simulink model "ACC_scenario_007_cs"  (Simulink 7.6)
settings: Solver=ode5  SolverMode=SingleTasking  StartTime=0.0  StopTime=inf  FixedStep=1/100  RelTol=1e-3
PreLoadFcn:
  | check_NUTS_defined;
  | 
  | assignin('base',['model_closed_' bdroot],false);addpath('../includes_ACC');
InitFcn:
  | PreScanExperimentInitFcn(bdroot)
StopFcn:
  | PreScanExperimentStopFcn(bdroot)
undefined symbols (not defined in the file): DiscIntNeverNeededParam, x_ini_VRML, y_ini_VRML, z_ini_VRML
libraries: PreScanSimulinkLibrary x47, simulink x27, PreScanUsefulBlocks x15, simulink_extras x13, simulink_need_slupdate x1
(part 3 of 25)

== system ACC_scenario_007_cs/HighwayOverhang_11 ==
  note: Resources
      |
      | 1: InformationSign\MatrixSign_GreenArrow.png: MatrixSign GreenArrow
      | 2: InformationSign\TASS_logo.jpeg: TASS logo
      | 3: InformationSign\SEARCH_Logo.png: SEARCH Logo
      | 4: InformationSign\TheFuture.jpg: TheFuture
      | 5: InformationSign\NoParking.jpg: NoParking
      | 6: InformationSign\dont_even_think.jpg: dont even think
      | 7: InformationSign\sharp_edges.jpg: sharp edges
      | 8: InformationSign\tacos.jpg: tacos
      | 9: InformationSign\cthulhuwarn.jpg: cthulhuwarn
      | 10: InformationSign\road_surprises.jpg: road surprises
      | 11: InformationSign\sign1.jpg: sign1
      | 12: InformationSign\sign2.jpg: sign2
      | 13: InformationSign\sign3.jpg: sign3
      | 14: InformationSign\sign4.jpg: sign4
      | 15: InformationSign\sign5.JPG: sign5
      | 16: InformationSign\sign7.JPG: sign7
      | 17: InformationSign\sign10.png: sign10
      | 18: InformationSign\sign31.jpg: sign31
      | 19: InformationSign\sign32.jpg: sign32
  note: Resources
  Ground_1 : Ground
  Ground_2 : Ground
  VISUMatrixSign_Mux : Subsystem  Tag=MuxMatrixSign
      settings: DeleteFcn=PreScanBlockDeleteFcn;
      mask Type: PreScan Mux Block:
  VISUMatrixSign_HighwayOverhang_11 : Outport  Tag=VisuMatrixSignOutport  SampleTime=1/100
  wires:
  VISUMatrixSign_Mux/1(MatrixSigns) -> VISUMatrixSign_HighwayOverhang_11/1
  Ground_1/1 -> VISUMatrixSign_Mux/1(InformationPlate_13)
  Ground_2/1 -> VISUMatrixSign_Mux/2(InformationPlate_14)

== system ACC_scenario_007_cs/HighwayOverhang_12 ==
  note: Resources
      |
      | 1: InformationSign\MatrixSign_GreenArrow.png: MatrixSign GreenArrow
      | 2: InformationSign\TASS_logo.jpeg: TASS logo
      | 3: InformationSign\SEARCH_Logo.png: SEARCH Logo
      | 4: InformationSign\TheFuture.jpg: TheFuture
      | 5: InformationSign\NoParking.jpg: NoParking
      | 6: InformationSign\dont_even_think.jpg: dont even think
      | 7: InformationSign\sharp_edges.jpg: sharp edges
      | 8: InformationSign\tacos.jpg: tacos
      | 9: InformationSign\cthulhuwarn.jpg: cthulhuwarn
      | 10: InformationSign\road_surprises.jpg: road surprises
      | 11: InformationSign\sign1.jpg: sign1
      | 12: InformationSign\sign2.jpg: sign2
      | 13: InformationSign\sign3.jpg: sign3
      | 14: InformationSign\sign4.jpg: sign4
      | 15: InformationSign\sign5.JPG: sign5
      | 16: InformationSign\sign7.JPG: sign7
      | 17: InformationSign\sign10.png: sign10
      | 18: InformationSign\sign31.jpg: sign31
      | 19: InformationSign\sign32.jpg: sign32
  note: Resources
  Ground_1 : Ground
  Ground_2 : Ground
  VISUMatrixSign_Mux : Subsystem  Tag=MuxMatrixSign
      settings: DeleteFcn=PreScanBlockDeleteFcn;
      mask Type: PreScan Mux Block:
  VISUMatrixSign_HighwayOverhang_12 : Outport  Tag=VisuMatrixSignOutport  SampleTime=1/100
  wires:
  VISUMatrixSign_Mux/1(MatrixSigns) -> VISUMatrixSign_HighwayOverhang_12/1
  Ground_1/1 -> VISUMatrixSign_Mux/1(InformationPlate_15)
  Ground_2/1 -> VISUMatrixSign_Mux/2(InformationPlate_16)

== system ACC_scenario_007_cs/HighwayOverhang_2 ==
  note: Resources
      |
      | 1: InformationSign\MatrixSign_GreenArrow.png: MatrixSign GreenArrow
      | 2: InformationSign\TASS_logo.jpeg: TASS logo
      | 3: InformationSign\SEARCH_Logo.png: SEARCH Logo
      | 4: InformationSign\TheFuture.jpg: TheFuture
      | 5: InformationSign\NoParking.jpg: NoParking
      | 6: InformationSign\dont_even_think.jpg: dont even think
      | 7: InformationSign\sharp_edges.jpg: sharp edges
      | 8: InformationSign\tacos.jpg: tacos
      | 9: InformationSign\cthulhuwarn.jpg: cthulhuwarn
      | 10: InformationSign\road_surprises.jpg: road surprises
      | 11: InformationSign\sign1.jpg: sign1
      | 12: InformationSign\sign2.jpg: sign2
      | 13: InformationSign\sign3.jpg: sign3
      | 14: InformationSign\sign4.jpg: sign4
      | 15: InformationSign\sign5.JPG: sign5
      | 16: InformationSign\sign7.JPG: sign7
      | 17: InformationSign\sign10.png: sign10
      | 18: InformationSign\sign31.jpg: sign31
      | 19: InformationSign\sign32.jpg: sign32
  note: Resources
  Ground_{1..4} (4 alike) : Ground
  VISUMatrixSignLights_Mux : Subsystem  Tag=MuxMatrixSignLights
      settings: DeleteFcn=PreScanBlockDeleteFcn;
      mask Type: PreScan Mux Block:
  VISUMatrixSign_Mux : Subsystem  Tag=MuxMatrixSign
      settings: DeleteFcn=PreScanBlockDeleteFcn;
      mask Type: PreScan Mux Block:
  VISUMatrixSign_HighwayOverhang_2 : Outport  Tag=VisuMatrixSignOutport  SampleTime=1/100
  VISUMatrixSignLights_HighwayOverhang_2 : Outport  Tag=VisuMatrixSignLightsOutport  Port=2  SampleTime=1/100
  wires:
  VISUMatrixSign_Mux/1(MatrixSigns) -> VISUMatrixSign_HighwayOverhang_2/1
  VISUMatrixSignLights_Mux/1(MatrixSignLights) -> VISUMatrixSignLights_HighwayOverhang_2/1
  Ground_1/1 -> VISUMatrixSignLights_Mux/1(MatrixSign_4)
  Ground_2/1 -> VISUMatrixSignLights_Mux/2(MatrixSign_5)
  Ground_3/1 -> VISUMatrixSign_Mux/1(MatrixSign_4)
  Ground_4/1 -> VISUMatrixSign_Mux/2(MatrixSign_5)

== system ACC_scenario_007_cs/HighwayOverhang_3 ==
  note: Resources
      |
      | 1: InformationSign\MatrixSign_GreenArrow.png: MatrixSign GreenArrow
      | 2: InformationSign\TASS_logo.jpeg: TASS logo
      | 3: InformationSign\SEARCH_Logo.png: SEARCH Logo
      | 4: InformationSign\TheFuture.jpg: TheFuture
      | 5: InformationSign\NoParking.jpg: NoParking
      | 6: InformationSign\dont_even_think.jpg: dont even think
      | 7: InformationSign\sharp_edges.jpg: sharp edges
      | 8: InformationSign\tacos.jpg: tacos
      | 9: InformationSign\cthulhuwarn.jpg: cthulhuwarn
      | 10: InformationSign\road_surprises.jpg: road surprises
      | 11: InformationSign\sign1.jpg: sign1
      | 12: InformationSign\sign2.jpg: sign2
      | 13: InformationSign\sign3.jpg: sign3
      | 14: InformationSign\sign4.jpg: sign4
      | 15: InformationSign\sign5.JPG: sign5
      | 16: InformationSign\sign7.JPG: sign7
      | 17: InformationSign\sign10.png: sign10
      | 18: InformationSign\sign31.jpg: sign31
      | 19: InformationSign\sign32.jpg: sign32
  note: Resources
  Ground_{1..4} (4 alike) : Ground
  VISUMatrixSignLights_Mux : Subsystem  Tag=MuxMatrixSignLights
      settings: DeleteFcn=PreScanBlockDeleteFcn;
      mask Type: PreScan Mux Block:
  VISUMatrixSign_Mux : Subsystem  Tag=MuxMatrixSign
      settings: DeleteFcn=PreScanBlockDeleteFcn;
      mask Type: PreScan Mux Block:
  VISUMatrixSign_HighwayOverhang_3 : Outport  Tag=VisuMatrixSignOutport  SampleTime=1/100
  VISUMatrixSignLights_HighwayOverhang_3 : Outport  Tag=VisuMatrixSignLightsOutport  Port=2  SampleTime=1/100
  wires:
  VISUMatrixSign_Mux/1(MatrixSigns) -> VISUMatrixSign_HighwayOverhang_3/1
  VISUMatrixSignLights_Mux/1(MatrixSignLights) -> VISUMatrixSignLights_HighwayOverhang_3/1
  Ground_1/1 -> VISUMatrixSignLights_Mux/1(MatrixSign_7)
  Ground_2/1 -> VISUMatrixSignLights_Mux/2(MatrixSign_8)
  Ground_3/1 -> VISUMatrixSign_Mux/1(MatrixSign_7)
  Ground_4/1 -> VISUMatrixSign_Mux/2(MatrixSign_8)

== system ACC_scenario_007_cs/HighwayOverhang_4 ==
  note: Resources
      |
      | 1: InformationSign\MatrixSign_GreenArrow.png: MatrixSign GreenArrow
      | 2: InformationSign\TASS_logo.jpeg: TASS logo
      | 3: InformationSign\SEARCH_Logo.png: SEARCH Logo
      | 4: InformationSign\TheFuture.jpg: TheFuture
      | 5: InformationSign\NoParking.jpg: NoParking
      | 6: InformationSign\dont_even_think.jpg: dont even think
      | 7: InformationSign\sharp_edges.jpg: sharp edges
      | 8: InformationSign\tacos.jpg: tacos
      | 9: InformationSign\cthulhuwarn.jpg: cthulhuwarn
      | 10: InformationSign\road_surprises.jpg: road surprises
      | 11: InformationSign\sign1.jpg: sign1
      | 12: InformationSign\sign2.jpg: sign2
      | 13: InformationSign\sign3.jpg: sign3
      | 14: InformationSign\sign4.jpg: sign4
      | 15: InformationSign\sign5.JPG: sign5
      | 16: InformationSign\sign7.JPG: sign7
      | 17: InformationSign\sign10.png: sign10
      | 18: InformationSign\sign31.jpg: sign31
      | 19: InformationSign\sign32.jpg: sign32
  note: Resources
  Ground_{1..4} (4 alike) : Ground
  VISUMatrixSignLights_Mux : Subsystem  Tag=MuxMatrixSignLights
      settings: DeleteFcn=PreScanBlockDeleteFcn;
      mask Type: PreScan Mux Block:
  VISUMatrixSign_Mux : Subsystem  Tag=MuxMatrixSign
      settings: DeleteFcn=PreScanBlockDeleteFcn;
      mask Type: PreScan Mux Block:
  VISUMatrixSign_HighwayOverhang_4 : Outport  Tag=VisuMatrixSignOutport  SampleTime=1/100
  VISUMatrixSignLights_HighwayOverhang_4 : Outport  Tag=VisuMatrixSignLightsOutport  Port=2  SampleTime=1/100
  wires:
  VISUMatrixSign_Mux/1(MatrixSigns) -> VISUMatrixSign_HighwayOverhang_4/1
  VISUMatrixSignLights_Mux/1(MatrixSignLights) -> VISUMatrixSignLights_HighwayOverhang_4/1
  Ground_1/1 -> VISUMatrixSignLights_Mux/1(MatrixSign_10)
  Ground_2/1 -> VISUMatrixSignLights_Mux/2(MatrixSign_11)
  Ground_3/1 -> VISUMatrixSign_Mux/1(MatrixSign_10)
  Ground_4/1 -> VISUMatrixSign_Mux/2(MatrixSign_11)

== system ACC_scenario_007_cs/HighwayOverhang_5 ==
  note: Resources
      |
      | 1: InformationSign\MatrixSign_GreenArrow.png: MatrixSign GreenArrow
      | 2: InformationSign\TASS_logo.jpeg: TASS logo
      | 3: InformationSign\SEARCH_Logo.png: SEARCH Logo
      | 4: InformationSign\TheFuture.jpg: TheFuture
      | 5: InformationSign\NoParking.jpg: NoParking
      | 6: InformationSign\dont_even_think.jpg: dont even think
      | 7: InformationSign\sharp_edges.jpg: sharp edges
      | 8: InformationSign\tacos.jpg: tacos
      | 9: InformationSign\cthulhuwarn.jpg: cthulhuwarn
      | 10: InformationSign\road_surprises.jpg: road surprises
      | 11: InformationSign\sign1.jpg: sign1
      | 12: InformationSign\sign2.jpg: sign2
      | 13: InformationSign\sign3.jpg: sign3
      | 14: InformationSign\sign4.jpg: sign4
      | 15: InformationSign\sign5.JPG: sign5
      | 16: InformationSign\sign7.JPG: sign7
      | 17: InformationSign\sign10.png: sign10
      | 18: InformationSign\sign31.jpg: sign31
      | 19: InformationSign\sign32.jpg: sign32
  note: Resources
  Ground_1 : Ground
  Ground_2 : Ground
  VISUMatrixSign_Mux : Subsystem  Tag=MuxMatrixSign
      settings: DeleteFcn=PreScanBlockDeleteFcn;
      mask Type: PreScan Mux Block:
  VISUMatrixSign_HighwayOverhang_5 : Outport  Tag=VisuMatrixSignOutport  SampleTime=1/100
  wires:
  VISUMatrixSign_Mux/1(MatrixSigns) -> VISUMatrixSign_HighwayOverhang_5/1
  Ground_1/1 -> VISUMatrixSign_Mux/1(InformationPlate_1)
  Ground_2/1 -> VISUMatrixSign_Mux/2(InformationPlate_2)

== system ACC_scenario_007_cs/HighwayOverhang_6 ==
  note: Resources
      |
      | 1: InformationSign\MatrixSign_GreenArrow.png: MatrixSign GreenArrow
      | 2: InformationSign\TASS_logo.jpeg: TASS logo
      | 3: InformationSign\SEARCH_Logo.png: SEARCH Logo
      | 4: InformationSign\TheFuture.jpg: TheFuture
      | 5: InformationSign\NoParking.jpg: NoParking
      | 6: InformationSign\dont_even_think.jpg: dont even think
      | 7: InformationSign\sharp_edges.jpg: sharp edges
      | 8: InformationSign\tacos.jpg: tacos
      | 9: InformationSign\cthulhuwarn.jpg: cthulhuwarn
      | 10: InformationSign\road_surprises.jpg: road surprises
      | 11: InformationSign\sign1.jpg: sign1
      | 12: InformationSign\sign2.jpg: sign2
      | 13: InformationSign\sign3.jpg: sign3
      | 14: InformationSign\sign4.jpg: sign4
      | 15: InformationSign\sign5.JPG: sign5
      | 16: InformationSign\sign7.JPG: sign7
      | 17: InformationSign\sign10.png: sign10
      | 18: InformationSign\sign31.jpg: sign31
      | 19: InformationSign\sign32.jpg: sign32
  note: Resources
  Ground_1 : Ground
  Ground_2 : Ground
  VISUMatrixSign_Mux : Subsystem  Tag=MuxMatrixSign
      settings: DeleteFcn=PreScanBlockDeleteFcn;
      mask Type: PreScan Mux Block:
  VISUMatrixSign_HighwayOverhang_6 : Outport  Tag=VisuMatrixSignOutport  SampleTime=1/100
  wires:
  VISUMatrixSign_Mux/1(MatrixSigns) -> VISUMatrixSign_HighwayOverhang_6/1
  Ground_1/1 -> VISUMatrixSign_Mux/1(InformationPlate_3)
  Ground_2/1 -> VISUMatrixSign_Mux/2(InformationPlate_4)

== system ACC_scenario_007_cs/HighwayOverhang_7 ==
  note: Resources
      |
      | 1: InformationSign\MatrixSign_GreenArrow.png: MatrixSign GreenArrow
      | 2: InformationSign\TASS_logo.jpeg: TASS logo
      | 3: InformationSign\SEARCH_Logo.png: SEARCH Logo
      | 4: InformationSign\TheFuture.jpg: TheFuture
      | 5: InformationSign\NoParking.jpg: NoParking
      | 6: InformationSign\dont_even_think.jpg: dont even think
      | 7: InformationSign\sharp_edges.jpg: sharp edges
      | 8: InformationSign\tacos.jpg: tacos
      | 9: InformationSign\cthulhuwarn.jpg: cthulhuwarn
      | 10: InformationSign\road_surprises.jpg: road surprises
      | 11: InformationSign\sign1.jpg: sign1
      | 12: InformationSign\sign2.jpg: sign2
      | 13: InformationSign\sign3.jpg: sign3
      | 14: InformationSign\sign4.jpg: sign4
      | 15: InformationSign\sign5.JPG: sign5
      | 16: InformationSign\sign7.JPG: sign7
      | 17: InformationSign\sign10.png: sign10
      | 18: InformationSign\sign31.jpg: sign31
      | 19: InformationSign\sign32.jpg: sign32
  note: Resources
  Ground_1 : Ground
  Ground_2 : Ground
  VISUMatrixSign_Mux : Subsystem  Tag=MuxMatrixSign
      settings: DeleteFcn=PreScanBlockDeleteFcn;
      mask Type: PreScan Mux Block:
  VISUMatrixSign_HighwayOverhang_7 : Outport  Tag=VisuMatrixSignOutport  SampleTime=1/100
  wires:
  VISUMatrixSign_Mux/1(MatrixSigns) -> VISUMatrixSign_HighwayOverhang_7/1
  Ground_1/1 -> VISUMatrixSign_Mux/1(InformationPlate_9)
  Ground_2/1 -> VISUMatrixSign_Mux/2(InformationPlate_10)

== system ACC_scenario_007_cs/HighwayOverhang_8 ==
  note: Resources
      |
      | 1: InformationSign\MatrixSign_GreenArrow.png: MatrixSign GreenArrow
      | 2: InformationSign\TASS_logo.jpeg: TASS logo
      | 3: InformationSign\SEARCH_Logo.png: SEARCH Logo
      | 4: InformationSign\TheFuture.jpg: TheFuture
      | 5: InformationSign\NoParking.jpg: NoParking
      | 6: InformationSign\dont_even_think.jpg: dont even think
      | 7: InformationSign\sharp_edges.jpg: sharp edges
      | 8: InformationSign\tacos.jpg: tacos
      | 9: InformationSign\cthulhuwarn.jpg: cthulhuwarn
      | 10: InformationSign\road_surprises.jpg: road surprises
      | 11: InformationSign\sign1.jpg: sign1
      | 12: InformationSign\sign2.jpg: sign2
      | 13: InformationSign\sign3.jpg: sign3
      | 14: InformationSign\sign4.jpg: sign4
      | 15: InformationSign\sign5.JPG: sign5
      | 16: InformationSign\sign7.JPG: sign7
      | 17: InformationSign\sign10.png: sign10
      | 18: InformationSign\sign31.jpg: sign31
      | 19: InformationSign\sign32.jpg: sign32
  note: Resources
  Ground_1 : Ground
  Ground_2 : Ground
  VISUMatrixSign_Mux : Subsystem  Tag=MuxMatrixSign
      settings: DeleteFcn=PreScanBlockDeleteFcn;
      mask Type: PreScan Mux Block:
  VISUMatrixSign_HighwayOverhang_8 : Outport  Tag=VisuMatrixSignOutport  SampleTime=1/100
  wires:
  VISUMatrixSign_Mux/1(MatrixSigns) -> VISUMatrixSign_HighwayOverhang_8/1
  Ground_1/1 -> VISUMatrixSign_Mux/1(InformationPlate_11)
  Ground_2/1 -> VISUMatrixSign_Mux/2(InformationPlate_12)

== system ACC_scenario_007_cs/HighwayOverhang_9 ==
  note: Resources
      |
      | 1: InformationSign\MatrixSign_GreenArrow.png: MatrixSign GreenArrow
      | 2: InformationSign\TASS_logo.jpeg: TASS logo
      | 3: InformationSign\SEARCH_Logo.png: SEARCH Logo
      | 4: InformationSign\TheFuture.jpg: TheFuture
      | 5: InformationSign\NoParking.jpg: NoParking
      | 6: InformationSign\dont_even_think.jpg: dont even think
      | 7: InformationSign\sharp_edges.jpg: sharp edges
      | 8: InformationSign\tacos.jpg: tacos
      | 9: InformationSign\cthulhuwarn.jpg: cthulhuwarn
      | 10: InformationSign\road_surprises.jpg: road surprises
      | 11: InformationSign\sign1.jpg: sign1
      | 12: InformationSign\sign2.jpg: sign2
      | 13: InformationSign\sign3.jpg: sign3
      | 14: InformationSign\sign4.jpg: sign4
      | 15: InformationSign\sign5.JPG: sign5
      | 16: InformationSign\sign7.JPG: sign7
      | 17: InformationSign\sign10.png: sign10
      | 18: InformationSign\sign31.jpg: sign31
      | 19: InformationSign\sign32.jpg: sign32
  note: Resources
  Ground_1 : Ground
  Ground_2 : Ground
  VISUMatrixSign_Mux : Subsystem  Tag=MuxMatrixSign
      settings: DeleteFcn=PreScanBlockDeleteFcn;
      mask Type: PreScan Mux Block:
  VISUMatrixSign_HighwayOverhang_9 : Outport  Tag=VisuMatrixSignOutport  SampleTime=1/100
  wires:
  VISUMatrixSign_Mux/1(MatrixSigns) -> VISUMatrixSign_HighwayOverhang_9/1
  Ground_1/1 -> VISUMatrixSign_Mux/1(InformationPlate_5)
  Ground_2/1 -> VISUMatrixSign_Mux/2(InformationPlate_6)

== system ACC_scenario_007_cs/Lightpost_1 ==

== system ACC_scenario_007_cs/Lightpost_19 ==

== system ACC_scenario_007_cs/Lightpost_2 ==

== system ACC_scenario_007_cs/Lightpost_21 ==

== system ACC_scenario_007_cs/Lightpost_23 ==

== system ACC_scenario_007_cs/Lightpost_24 ==

== system ACC_scenario_007_cs/Lightpost_25 ==

== system ACC_scenario_007_cs/Lightpost_26 ==

== system ACC_scenario_007_cs/Lightpost_27 ==

== system ACC_scenario_007_cs/Lightpost_28 ==

== system ACC_scenario_007_cs/Lightpost_29 ==

== system ACC_scenario_007_cs/Lightpost_3 ==

== system ACC_scenario_007_cs/Lightpost_30 ==

== system ACC_scenario_007_cs/Lightpost_31 ==

== system ACC_scenario_007_cs/Lightpost_32 ==

== system ACC_scenario_007_cs/Lightpost_33 ==

== system ACC_scenario_007_cs/Lightpost_34 ==

== system ACC_scenario_007_cs/Lightpost_35 ==

== system ACC_scenario_007_cs/Lightpost_36 ==

== system ACC_scenario_007_cs/Lightpost_37 ==

== system ACC_scenario_007_cs/Lightpost_4 ==

== system ACC_scenario_007_cs/Lightpost_52 ==

== system ACC_scenario_007_cs/Lightpost_53 ==

== system ACC_scenario_007_cs/Lightpost_54 ==

== system ACC_scenario_007_cs/Lightpost_55 ==

== system ACC_scenario_007_cs/Lightpost_56 ==

== system ACC_scenario_007_cs/Lightpost_57 ==

== system ACC_scenario_007_cs/Lightpost_58 ==

== system ACC_scenario_007_cs/Lightpost_59 ==

== system ACC_scenario_007_cs/Lightpost_60 ==

== system ACC_scenario_007_cs/Lightpost_61 ==

== system ACC_scenario_007_cs/Lightpost_62 ==

== system ACC_scenario_007_cs/Lightpost_63 ==

== system ACC_scenario_007_cs/Lightpost_64 ==

== system ACC_scenario_007_cs/Lightpost_65 ==

== system ACC_scenario_007_cs/Lightpost_66 ==

== system ACC_scenario_007_cs/Lightpost_67 ==

== system ACC_scenario_007_cs/Lightpost_68 ==

== system ACC_scenario_007_cs/Nissan_Cabstar_1 ==
  SELF_Nissan_Cabstar_1 : Inport  Tag=SELFInport
  Ground_{1..20} (20 alike) : Ground
  SELF_Demux : Subsystem  Tag=DemuxSELF
      settings: DeleteFcn=PreScanBlockDeleteFcn;
      mask Type: PreScan Demux Block:
      mask Display:
      | port_label('output',1,'x [m]')
      | port_label('output',2,'y [m]')
      | port_label('output',3,'z [m]','texmode','on')
      | port_label('output',4,'Rotx [deg]','texmode','on')
      | port_label('output',5,'Roty [deg]','texmode','on')
      | port_label('output',6,'Rotz [deg]','texmode','on')
      | port_label('output',7,'GPS lat [deg]','texmode','on')
      | port_label('output',8,'GPS long [deg]','texmode','on')
      | port_label('output',9,'GPS alt [m]','texmode','on')
      | port_label('output',10,'Velocity [m/s]')
      | port_label('output',11,'Heading [deg]')
      | port_label('output',12,'Yaw rate [deg/s]')
  Terminator_{1..11} (11 alike) : Terminator
  Trajectories : Subsystem  Tag=Trajectories
      settings: DeleteFcn=PreScanBlockDeleteFcn;
      mask Type: PreScan Source Block: Trajectories
      mask Description:
      | The Trajectory block contains the trajectories defined in the PreScan Experiment Editor. The output of the trajectory block is the (vehicle) State.
      | 
      | For more information, please click Help.
      mask Help: eval('openHelp(312)')
      mask Display: image(imread('Trajectory.png'));
  VISULightSource_Mux : Subsystem  Tag=MuxLightSource
      settings: DeleteFcn=PreScanBlockDeleteFcn;
      mask Type: PreScan Mux Block:
  VISUSteer_Mux : Subsystem  Tag=MuxSteer
      settings: DeleteFcn=PreScanBlockDeleteFcn;
      mask Type: PreScan Mux Block:
  VISUWheels_Mux : Subsystem  Tag=MuxWheels  mask(PreScan Mux Block:){TimeStep=1/100}
      mask prompts: TimeStep "Auto Assigned: Time Step [s]"
      settings: DeleteFcn=PreScanBlockDeleteFcn;
  STATE_Nissan_Cabstar_1 : Outport  Tag=StateOutport
  VISULightSource_Nissan_Cabstar_1 : Outport  Tag=VisuLightsOutport  Port=2  SampleTime=1/100
  VISUSteer_Nissan_Cabstar_1 : Outport  Tag=VisuSteerOutport  Port=3  SampleTime=1/100
  VISUWheels_Nissan_Cabstar_1 : Outport  Tag=VisuWheelsOutport  Port=4  SampleTime=1/100
  wires:
  SELF_Nissan_Cabstar_1/1 -> SELF_Demux/1(SELF)
  VISULightSource_Mux/1(Lights) -> VISULightSource_Nissan_Cabstar_1/1
  VISUSteer_Mux/1(Steer) -> VISUSteer_Nissan_Cabstar_1/1
  VISUWheels_Mux/1(Wheels) -> VISUWheels_Nissan_Cabstar_1/1
  Trajectories/1(State) -> STATE_Nissan_Cabstar_1/1
  SELF_Demux/10(velocity [m/s]) -> VISUWheels_Mux/3(Velocity [m/s])
  SELF_Demux/1(x [m]) -> Terminator_1/1
  SELF_Demux/2(y [m]) -> Terminator_2/1
  SELF_Demux/3(z [m]) -> Terminator_3/1
  SELF_Demux/4(rotx [deg]) -> Terminator_4/1
  SELF_Demux/5(roty [deg]) -> Terminator_5/1
  SELF_Demux/6(rotz [deg]) -> Terminator_6/1
  SELF_Demux/7(GPS lat [deg]) -> Terminator_7/1
  SELF_Demux/8(GPS long [deg]) -> Terminator_8/1
  SELF_Demux/9(GPS alt [m]) -> Terminator_9/1
  SELF_Demux/11(heading [deg]) -> Terminator_10/1
  SELF_Demux/12(yaw rate [deg/s]) -> Terminator_11/1
  Ground_1/1 -> VISULightSource_Mux/1(IndicatorLightsLeft)
  Ground_2/1 -> VISULightSource_Mux/2(IndicatorLightsRight)
  Ground_3/1 -> VISULightSource_Mux/3(FogLightRearLeft)
  Ground_4/1 -> VISULightSource_Mux/4(FogLightRearRight)
  Ground_5/1 -> VISULightSource_Mux/5(FogLightFrontLeft)
  Ground_6/1 -> VISULightSource_Mux/6(FogLightFrontRight)
  Ground_7/1 -> VISULightSource_Mux/7(MainLightRearLeft)
  Ground_8/1 -> VISULightSource_Mux/8(MainLightRearRight)
  Ground_9/1 -> VISULightSource_Mux/9(MainLightFrontLeft)
  Ground_10/1 -> VISULightSource_Mux/10(MainLightFrontRight)
  Ground_11/1 -> VISULightSource_Mux/11(BrakeLightLeft)
  Ground_12/1 -> VISULightSource_Mux/12(BrakeLightRight)
  Ground_13/1 -> VISUSteer_Mux/1(Column [deg])
  Ground_14/1 -> VISUSteer_Mux/2(Steer [deg])
  Ground_15/1 -> VISUWheels_Mux/1(Steer Angle Front [deg])
  Ground_16/1 -> VISUWheels_Mux/2(Steer Angle Rear [deg])
  Ground_17/1 -> VISUWheels_Mux/4(zDisp FL [m])
  Ground_18/1 -> VISUWheels_Mux/5(zDisp FR [m])
  Ground_19/1 -> VISUWheels_Mux/6(zDisp RL [m])
  Ground_20/1 -> VISUWheels_Mux/7(zDisp RR [m])

== system ACC_scenario_007_cs/SELF_Actros_1860_1 ==
  Selector : Selector  IndexMode=Zero-based  IndexOptions=Starting index (dialog)  Indices=PortIndex  OutputSizes=PortWidth(1)
  U : Outport
  wires:
  Selector/1 -> U/1
  [SimCoreSensors] -> Selector/1

== system ACC_scenario_007_cs/SELF_Actros_2541_1 ==
  Selector : Selector  IndexMode=Zero-based  IndexOptions=Starting index (dialog)  Indices=PortIndex  OutputSizes=PortWidth(1)
  U : Outport
  wires:
  Selector/1 -> U/1
  [SimCoreSensors] -> Selector/1

== system ACC_scenario_007_cs/SELF_Actros_2541_2 ==
  Selector : Selector  IndexMode=Zero-based  IndexOptions=Starting index (dialog)  Indices=PortIndex  OutputSizes=PortWidth(1)
  U : Outport
  wires:
  Selector/1 -> U/1
  [SimCoreSensors] -> Selector/1

== system ACC_scenario_007_cs/SELF_Actros_2541_3 ==
  Selector : Selector  IndexMode=Zero-based  IndexOptions=Starting index (dialog)  Indices=PortIndex  OutputSizes=PortWidth(1)
  U : Outport
  wires:
  Selector/1 -> U/1
  [SimCoreSensors] -> Selector/1

== system ACC_scenario_007_cs/SELF_Audi_A8_1 ==
  Selector : Selector  IndexMode=Zero-based  IndexOptions=Starting index (dialog)  Indices=PortIndex  OutputSizes=PortWidth(1)
  U : Outport
  wires:
  Selector/1 -> U/1
  [SimCoreSensors] -> Selector/1

== system ACC_scenario_007_cs/SELF_BMW_X5_1 ==
  Selector : Selector  IndexMode=Zero-based  IndexOptions=Starting index (dialog)  Indices=PortIndex  OutputSizes=PortWidth(1)
  U : Outport
  wires:
  Selector/1 -> U/1
  [SimCoreSensors] -> Selector/1

== system ACC_scenario_007_cs/SELF_BMW_X5_2 ==
  Selector : Selector  IndexMode=Zero-based  IndexOptions=Starting index (dialog)  Indices=PortIndex  OutputSizes=PortWidth(1)
  U : Outport
  wires:
  Selector/1 -> U/1
  [SimCoreSensors] -> Selector/1

== system ACC_scenario_007_cs/SELF_Citroen_C3_1 ==
  Selector : Selector  IndexMode=Zero-based  IndexOptions=Starting index (dialog)  Indices=PortIndex  OutputSizes=PortWidth(1)
  U : Outport
  wires:
  Selector/1 -> U/1
  [SimCoreSensors] -> Selector/1

== system ACC_scenario_007_cs/SELF_ContainerTrailer_DoubleAxle_1 ==
  Selector : Selector  IndexMode=Zero-based  IndexOptions=Starting index (dialog)  Indices=PortIndex  OutputSizes=PortWidth(1)
  U : Outport
  wires:
  Selector/1 -> U/1
  [SimCoreSensors] -> Selector/1

== system ACC_scenario_007_cs/SELF_Fiat_Bravo_1 ==
  Selector : Selector  IndexMode=Zero-based  IndexOptions=Starting index (dialog)  Indices=PortIndex  OutputSizes=PortWidth(1)
  U : Outport
  wires:
  Selector/1 -> U/1
  [SimCoreSensors] -> Selector/1

== system ACC_scenario_007_cs/SELF_Nissan_Cabstar_1 ==
  Selector : Selector  IndexMode=Zero-based  IndexOptions=Starting index (dialog)  Indices=PortIndex  OutputSizes=PortWidth(1)
  U : Outport
  wires:
  Selector/1 -> U/1
  [SimCoreSensors] -> Selector/1

== system ACC_scenario_007_cs/SELF_Sphere_1 ==
  Selector : Selector  IndexMode=Zero-based  IndexOptions=Starting index (dialog)  Indices=PortIndex  OutputSizes=PortWidth(1)
  U : Outport
  wires:
  Selector/1 -> U/1
  [SimCoreSensors] -> Selector/1

== system ACC_scenario_007_cs/SELF_Toyota_Yaris_1 ==
  Selector : Selector  IndexMode=Zero-based  IndexOptions=Starting index (dialog)  Indices=PortIndex  OutputSizes=PortWidth(1)
  U : Outport
  wires:
  Selector/1 -> U/1
  [SimCoreSensors] -> Selector/1

== system ACC_scenario_007_cs/STATE_Actros_1860_1 ==
  State : Inport  SampleTime=TimeStep
  Acceleration : Subsystem  mask(ToSensorWorld){PortName=Acceleration_Actros_1860_1, PortIndex=1240, PortWidth=3, TimeStep=1/100}
      mask prompts: PortName "Port Name [-]"; PortIndex "Index [-]"; PortWidth "Port Dimensions [-]"; TimeStep "Upload Period [s]"
      mask help: Sends signal(s) from Simulink to the Sensor World
      mask Help:
      | Sends signal(s) from Simulink to the Sensor World.
      | 
      | - Port Name [-]: specifies the name of the output terminal of the Simulink block.
      | - Index [-]: specifies the start position in the output vector sent to the Sensor World
      | - Port dimensions [-]: specifies the width (number of scalars) of the output port
      | - Upload period [s]: specifies the upload period (in seconds) of the port.
      mask Display: port_label('input', 1, PortName)
  Demux : Demux  Outputs=5
  Position : Subsystem  mask(ToSensorWorld){PortName=Position_Actros_1860_1, PortIndex=1234, PortWidth=3, TimeStep=1/100}
      mask prompts: PortName "Port Name [-]"; PortIndex "Index [-]"; PortWidth "Port Dimensions [-]"; TimeStep "Upload Period [s]"
      mask help: Sends signal(s) from Simulink to the Sensor World
      mask Help:
      | Sends signal(s) from Simulink to the Sensor World.
      | 
      | - Port Name [-]: specifies the name of the output terminal of the Simulink block.
      | - Index [-]: specifies the start position in the output vector sent to the Sensor World
      | - Port dimensions [-]: specifies the width (number of scalars) of the output port
      | - Upload period [s]: specifies the upload period (in seconds) of the port.
      mask Display: port_label('input', 1, PortName)
  RPY : Subsystem  mask(ToSensorWorld){PortName=RPY_Actros_1860_1, PortIndex=1243, PortWidth=3, TimeStep=1/100}
      mask prompts: PortName "Port Name [-]"; PortIndex "Index [-]"; PortWidth "Port Dimensions [-]"; TimeStep "Upload Period [s]"
      mask help: Sends signal(s) from Simulink to the Sensor World
      mask Help:
      | Sends signal(s) from Simulink to the Sensor World.
      | 
      | - Port Name [-]: specifies the name of the output terminal of the Simulink block.
      | - Index [-]: specifies the start position in the output vector sent to the Sensor World
      | - Port dimensions [-]: specifies the width (number of scalars) of the output port
      | - Upload period [s]: specifies the upload period (in seconds) of the port.
      mask Display: port_label('input', 1, PortName)
  Velocity : Subsystem  mask(ToSensorWorld){PortName=Velocity_Actros_1860_1, PortIndex=1237, PortWidth=3, TimeStep=1/100}
      mask prompts: PortName "Port Name [-]"; PortIndex "Index [-]"; PortWidth "Port Dimensions [-]"; TimeStep "Upload Period [s]"
      mask help: Sends signal(s) from Simulink to the Sensor World
      mask Help:
      | Sends signal(s) from Simulink to the Sensor World.
      | 
      | - Port Name [-]: specifies the name of the output terminal of the Simulink block.
      | - Index [-]: specifies the start position in the output vector sent to the Sensor World
      | - Port dimensions [-]: specifies the width (number of scalars) of the output port
      | - Upload period [s]: specifies the upload period (in seconds) of the port.
      mask Display: port_label('input', 1, PortName)
  iniBODYAngles wrtVEH [rad] : Constant  Value=[Roll0 Pitch0 0]  SampleTime=TimeStep
  iniVEHAngles wrtGLOB [rad] : Constant  Value=[0 0 Yaw0]  SampleTime=TimeStep
  iniVEHposition wrtGLOB [m] : Constant  Value=[x0 y0 z0]  SampleTime=TimeStep
  state2FullState : PreScanSimulinkLibrary/state2FullState  SystemSampleTime=-1
  velRPY : Subsystem  mask(ToSensorWorld){PortName=velRPY_Actros_1860_1, PortIndex=1246, PortWidth=3, TimeStep=1/100}
      mask prompts: PortName "Port Name [-]"; PortIndex "Index [-]"; PortWidth "Port Dimensions [-]"; TimeStep "Upload Period [s]"
      mask help: Sends signal(s) from Simulink to the Sensor World
      mask Help:
      | Sends signal(s) from Simulink to the Sensor World.
      | 
      | - Port Name [-]: specifies the name of the output terminal of the Simulink block.
      | - Index [-]: specifies the start position in the output vector sent to the Sensor World
      | - Port dimensions [-]: specifies the width (number of scalars) of the output port
      | - Upload period [s]: specifies the upload period (in seconds) of the port.
      mask Display: port_label('input', 1, PortName)
  wires:
  Demux/5 -> velRPY/1(Y)
  Demux/4 -> RPY/1(Y)
  Demux/3 -> Acceleration/1(Y)
  Demux/2 -> Velocity/1(Y)
  Demux/1 -> Position/1(Y)
  State/1 -> state2FullState/1
  state2FullState/1 -> Demux/1
  iniVEHposition wrtGLOB [m]/1 -> state2FullState/2
  iniBODYAngles wrtVEH [rad]/1 -> state2FullState/3
  iniVEHAngles wrtGLOB [rad]/1 -> state2FullState/4

== system ACC_scenario_007_cs/STATE_Actros_2541_1 ==
  State : Inport  SampleTime=TimeStep
  Acceleration : Subsystem  mask(ToSensorWorld){PortName=Acceleration_Actros_2541_1, PortIndex=993, PortWidth=3, TimeStep=1/100}
      mask prompts: PortName "Port Name [-]"; PortIndex "Index [-]"; PortWidth "Port Dimensions [-]"; TimeStep "Upload Period [s]"
      mask help: Sends signal(s) from Simulink to the Sensor World
      mask Help:
      | Sends signal(s) from Simulink to the Sensor World.
      | 
      | - Port Name [-]: specifies the name of the output terminal of the Simulink block.
      | - Index [-]: specifies the start position in the output vector sent to the Sensor World
      | - Port dimensions [-]: specifies the width (number of scalars) of the output port
      | - Upload period [s]: specifies the upload period (in seconds) of the port.
      mask Display: port_label('input', 1, PortName)
  Demux : Demux  Outputs=5
  Position : Subsystem  mask(ToSensorWorld){PortName=Position_Actros_2541_1, PortIndex=987, PortWidth=3, TimeStep=1/100}
      mask prompts: PortName "Port Name [-]"; PortIndex "Index [-]"; PortWidth "Port Dimensions [-]"; TimeStep "Upload Period [s]"
      mask help: Sends signal(s) from Simulink to the Sensor World
      mask Help:
      | Sends signal(s) from Simulink to the Sensor World.
      | 
      | - Port Name [-]: specifies the name of the output terminal of the Simulink block.
      | - Index [-]: specifies the start position in the output vector sent to the Sensor World
      | - Port dimensions [-]: specifies the width (number of scalars) of the output port
      | - Upload period [s]: specifies the upload period (in seconds) of the port.
      mask Display: port_label('input', 1, PortName)
  RPY : Subsystem  mask(ToSensorWorld){PortName=RPY_Actros_2541_1, PortIndex=996, PortWidth=3, TimeStep=1/100}
      mask prompts: PortName "Port Name [-]"; PortIndex "Index [-]"; PortWidth "Port Dimensions [-]"; TimeStep "Upload Period [s]"
      mask help: Sends signal(s) from Simulink to the Sensor World
      mask Help:
      | Sends signal(s) from Simulink to the Sensor World.
      | 
      | - Port Name [-]: specifies the name of the output terminal of the Simulink block.
      | - Index [-]: specifies the start position in the output vector sent to the Sensor World
      | - Port dimensions [-]: specifies the width (number of scalars) of the output port
      | - Upload period [s]: specifies the upload period (in seconds) of the port.
      mask Display: port_label('input', 1, PortName)
  Velocity : Subsystem  mask(ToSensorWorld){PortName=Velocity_Actros_2541_1, PortIndex=990, PortWidth=3, TimeStep=1/100}
      mask prompts: PortName "Port Name [-]"; PortIndex "Index [-]"; PortWidth "Port Dimensions [-]"; TimeStep "Upload Period [s]"
      mask help: Sends signal(s) from Simulink to the Sensor World
      mask Help:
      | Sends signal(s) from Simulink to the Sensor World.
      | 
      | - Port Name [-]: specifies the name of the output terminal of the Simulink block.
      | - Index [-]: specifies the start position in the output vector sent to the Sensor World
      | - Port dimensions [-]: specifies the width (number of scalars) of the output port
      | - Upload period [s]: specifies the upload period (in seconds) of the port.
      mask Display: port_label('input', 1, PortName)
  iniBODYAngles wrtVEH [rad] : Constant  Value=[Roll0 Pitch0 0]  SampleTime=TimeStep
  iniVEHAngles wrtGLOB [rad] : Constant  Value=[0 0 Yaw0]  SampleTime=TimeStep
  iniVEHposition wrtGLOB [m] : Constant  Value=[x0 y0 z0]  SampleTime=TimeStep
  state2FullState : PreScanSimulinkLibrary/state2FullState  SystemSampleTime=-1
  velRPY : Subsystem  mask(ToSensorWorld){PortName=velRPY_Actros_2541_1, PortIndex=999, PortWidth=3, TimeStep=1/100}
      mask prompts: PortName "Port Name [-]"; PortIndex "Index [-]"; PortWidth "Port Dimensions [-]"; TimeStep "Upload Period [s]"
      mask help: Sends signal(s) from Simulink to the Sensor World
      mask Help:
      | Sends signal(s) from Simulink to the Sensor World.
      | 
      | - Port Name [-]: specifies the name of the output terminal of the Simulink block.
      | - Index [-]: specifies the start position in the output vector sent to the Sensor World
      | - Port dimensions [-]: specifies the width (number of scalars) of the output port
      | - Upload period [s]: specifies the upload period (in seconds) of the port.
      mask Display: port_label('input', 1, PortName)
  wires:
  Demux/5 -> velRPY/1(Y)
  Demux/4 -> RPY/1(Y)
  Demux/3 -> Acceleration/1(Y)
  Demux/2 -> Velocity/1(Y)
  Demux/1 -> Position/1(Y)
  State/1 -> state2FullState/1
  state2FullState/1 -> Demux/1
  iniVEHposition wrtGLOB [m]/1 -> state2FullState/2
  iniBODYAngles wrtVEH [rad]/1 -> state2FullState/3
  iniVEHAngles wrtGLOB [rad]/1 -> state2FullState/4

== system ACC_scenario_007_cs/STATE_Actros_2541_2 ==
  State : Inport  SampleTime=TimeStep
  Acceleration : Subsystem  mask(ToSensorWorld){PortName=Acceleration_Actros_2541_2, PortIndex=2215, PortWidth=3, TimeStep=1/100}
      mask prompts: PortName "Port Name [-]"; PortIndex "Index [-]"; PortWidth "Port Dimensions [-]"; TimeStep "Upload Period [s]"
      mask help: Sends signal(s) from Simulink to the Sensor World
      mask Help:
      | Sends signal(s) from Simulink to the Sensor World.
      | 
      | - Port Name [-]: specifies the name of the output terminal of the Simulink block.
      | - Index [-]: specifies the start position in the output vector sent to the Sensor World
      | - Port dimensions [-]: specifies the width (number of scalars) of the output port
      | - Upload period [s]: specifies the upload period (in seconds) of the port.
      mask Display: port_label('input', 1, PortName)
  Demux : Demux  Outputs=5
  Position : Subsystem  mask(ToSensorWorld){PortName=Position_Actros_2541_2, PortIndex=2209, PortWidth=3, TimeStep=1/100}
      mask prompts: PortName "Port Name [-]"; PortIndex "Index [-]"; PortWidth "Port Dimensions [-]"; TimeStep "Upload Period [s]"
      mask help: Sends signal(s) from Simulink to the Sensor World
      mask Help:
      | Sends signal(s) from Simulink to the Sensor World.
      | 
      | - Port Name [-]: specifies the name of the output terminal of the Simulink block.
      | - Index [-]: specifies the start position in the output vector sent to the Sensor World
      | - Port dimensions [-]: specifies the width (number of scalars) of the output port
      | - Upload period [s]: specifies the upload period (in seconds) of the port.
      mask Display: port_label('input', 1, PortName)
  RPY : Subsystem  mask(ToSensorWorld){PortName=RPY_Actros_2541_2, PortIndex=2218, PortWidth=3, TimeStep=1/100}
      mask prompts: PortName "Port Name [-]"; PortIndex "Index [-]"; PortWidth "Port Dimensions [-]"; TimeStep "Upload Period [s]"
      mask help: Sends signal(s) from Simulink to the Sensor World
      mask Help:
      | Sends signal(s) from Simulink to the Sensor World.
      | 
      | - Port Name [-]: specifies the name of the output terminal of the Simulink block.
      | - Index [-]: specifies the start position in the output vector sent to the Sensor World
      | - Port dimensions [-]: specifies the width (number of scalars) of the output port
      | - Upload period [s]: specifies the upload period (in seconds) of the port.
      mask Display: port_label('input', 1, PortName)
  Velocity : Subsystem  mask(ToSensorWorld){PortName=Velocity_Actros_2541_2, PortIndex=2212, PortWidth=3, TimeStep=1/100}
      mask prompts: PortName "Port Name [-]"; PortIndex "Index [-]"; PortWidth "Port Dimensions [-]"; TimeStep "Upload Period [s]"
      mask help: Sends signal(s) from Simulink to the Sensor World
      mask Help:
      | Sends signal(s) from Simulink to the Sensor World.
      | 
      | - Port Name [-]: specifies the name of the output terminal of the Simulink block.
      | - Index [-]: specifies the start position in the output vector sent to the Sensor World
      | - Port dimensions [-]: specifies the width (number of scalars) of the output port
      | - Upload period [s]: specifies the upload period (in seconds) of the port.
      mask Display: port_label('input', 1, PortName)
  iniBODYAngles wrtVEH [rad] : Constant  Value=[Roll0 Pitch0 0]  SampleTime=TimeStep
  iniVEHAngles wrtGLOB [rad] : Constant  Value=[0 0 Yaw0]  SampleTime=TimeStep
  iniVEHposition wrtGLOB [m] : Constant  Value=[x0 y0 z0]  SampleTime=TimeStep
  state2FullState : PreScanSimulinkLibrary/state2FullState  SystemSampleTime=-1
  velRPY : Subsystem  mask(ToSensorWorld){PortName=velRPY_Actros_2541_2, PortIndex=2221, PortWidth=3, TimeStep=1/100}
      mask prompts: PortName "Port Name [-]"; PortIndex "Index [-]"; PortWidth "Port Dimensions [-]"; TimeStep "Upload Period [s]"
      mask help: Sends signal(s) from Simulink to the Sensor World
      mask Help:
      | Sends signal(s) from Simulink to the Sensor World.
      | 
      | - Port Name [-]: specifies the name of the output terminal of the Simulink block.
      | - Index [-]: specifies the start position in the output vector sent to the Sensor World
      | - Port dimensions [-]: specifies the width (number of scalars) of the output port
      | - Upload period [s]: specifies the upload period (in seconds) of the port.
      mask Display: port_label('input', 1, PortName)
  wires:
  Demux/5 -> velRPY/1(Y)
  Demux/4 -> RPY/1(Y)
  Demux/3 -> Acceleration/1(Y)
  Demux/2 -> Velocity/1(Y)
  Demux/1 -> Position/1(Y)
  State/1 -> state2FullState/1
  state2FullState/1 -> Demux/1
  iniVEHposition wrtGLOB [m]/1 -> state2FullState/2
  iniBODYAngles wrtVEH [rad]/1 -> state2FullState/3
  iniVEHAngles wrtGLOB [rad]/1 -> state2FullState/4

== system ACC_scenario_007_cs/STATE_Actros_2541_3 ==
  State : Inport  SampleTime=TimeStep
  Acceleration : Subsystem  mask(ToSensorWorld){PortName=Acceleration_Actros_2541_3, PortIndex=2230, PortWidth=3, TimeStep=1/100}
      mask prompts: PortName "Port Name [-]"; PortIndex "Index [-]"; PortWidth "Port Dimensions [-]"; TimeStep "Upload Period [s]"
      mask help: Sends signal(s) from Simulink to the Sensor World
      mask Help:
      | Sends signal(s) from Simulink to the Sensor World.
      | 
      | - Port Name [-]: specifies the name of the output terminal of the Simulink block.
      | - Index [-]: specifies the start position in the output vector sent to the Sensor World
      | - Port dimensions [-]: specifies the width (number of scalars) of the output port
      | - Upload period [s]: specifies the upload period (in seconds) of the port.
      mask Display: port_label('input', 1, PortName)
  Demux : Demux  Outputs=5
  Position : Subsystem  mask(ToSensorWorld){PortName=Position_Actros_2541_3, PortIndex=2224, PortWidth=3, TimeStep=1/100}
      mask prompts: PortName "Port Name [-]"; PortIndex "Index [-]"; PortWidth "Port Dimensions [-]"; TimeStep "Upload Period [s]"
      mask help: Sends signal(s) from Simulink to the Sensor World
      mask Help:
      | Sends signal(s) from Simulink to the Sensor World.
      | 
      | - Port Name [-]: specifies the name of the output terminal of the Simulink block.
      | - Index [-]: specifies the start position in the output vector sent to the Sensor World
      | - Port dimensions [-]: specifies the width (number of scalars) of the output port
      | - Upload period [s]: specifies the upload period (in seconds) of the port.
      mask Display: port_label('input', 1, PortName)
  RPY : Subsystem  mask(ToSensorWorld){PortName=RPY_Actros_2541_3, PortIndex=2233, PortWidth=3, TimeStep=1/100}
      mask prompts: PortName "Port Name [-]"; PortIndex "Index [-]"; PortWidth "Port Dimensions [-]"; TimeStep "Upload Period [s]"
      mask help: Sends signal(s) from Simulink to the Sensor World
      mask Help:
      | Sends signal(s) from Simulink to the Sensor World.
      | 
      | - Port Name [-]: specifies the name of the output terminal of the Simulink block.
      | - Index [-]: specifies the start position in the output vector sent to the Sensor World
      | - Port dimensions [-]: specifies the width (number of scalars) of the output port
      | - Upload period [s]: specifies the upload period (in seconds) of the port.
      mask Display: port_label('input', 1, PortName)
  Velocity : Subsystem  mask(ToSensorWorld){PortName=Velocity_Actros_2541_3, PortIndex=2227, PortWidth=3, TimeStep=1/100}
      mask prompts: PortName "Port Name [-]"; PortIndex "Index [-]"; PortWidth "Port Dimensions [-]"; TimeStep "Upload Period [s]"
      mask help: Sends signal(s) from Simulink to the Sensor World
      mask Help:
      | Sends signal(s) from Simulink to the Sensor World.
      | 
      | - Port Name [-]: specifies the name of the output terminal of the Simulink block.
      | - Index [-]: specifies the start position in the output vector sent to the Sensor World
      | - Port dimensions [-]: specifies the width (number of scalars) of the output port
      | - Upload period [s]: specifies the upload period (in seconds) of the port.
      mask Display: port_label('input', 1, PortName)
  iniBODYAngles wrtVEH [rad] : Constant  Value=[Roll0 Pitch0 0]  SampleTime=TimeStep
  iniVEHAngles wrtGLOB [rad] : Constant  Value=[0 0 Yaw0]  SampleTime=TimeStep
  iniVEHposition wrtGLOB [m] : Constant  Value=[x0 y0 z0]  SampleTime=TimeStep
  state2FullState : PreScanSimulinkLibrary/state2FullState  SystemSampleTime=-1
  velRPY : Subsystem  mask(ToSensorWorld){PortName=velRPY_Actros_2541_3, PortIndex=2236, PortWidth=3, TimeStep=1/100}
      mask prompts: PortName "Port Name [-]"; PortIndex "Index [-]"; PortWidth "Port Dimensions [-]"; TimeStep "Upload Period [s]"
      mask help: Sends signal(s) from Simulink to the Sensor World
      mask Help:
      | Sends signal(s) from Simulink to the Sensor World.
      | 
      | - Port Name [-]: specifies the name of the output terminal of the Simulink block.
      | - Index [-]: specifies the start position in the output vector sent to the Sensor World
      | - Port dimensions [-]: specifies the width (number of scalars) of the output port
      | - Upload period [s]: specifies the upload period (in seconds) of the port.
      mask Display: port_label('input', 1, PortName)
  wires:
  Demux/5 -> velRPY/1(Y)
  Demux/4 -> RPY/1(Y)
  Demux/3 -> Acceleration/1(Y)
  Demux/2 -> Velocity/1(Y)
  Demux/1 -> Position/1(Y)
  State/1 -> state2FullState/1
  state2FullState/1 -> Demux/1
  iniVEHposition wrtGLOB [m]/1 -> state2FullState/2
  iniBODYAngles wrtVEH [rad]/1 -> state2FullState/3
  iniVEHAngles wrtGLOB [rad]/1 -> state2FullState/4
